simulink model "Final_V2"  (R2024a)
settings: Solver=FixedStepAuto  StartTime=0.0  StopTime=inf  FixedStep=1e-3  RelTol=1e-3
libraries: fl_lib x107, nesl_utility x75, ee_lib x16, batteryecm_lib x14, simulink x2
(part 1 of 16)
settings Model: Creator=DELL  LastModifiedBy=DELL

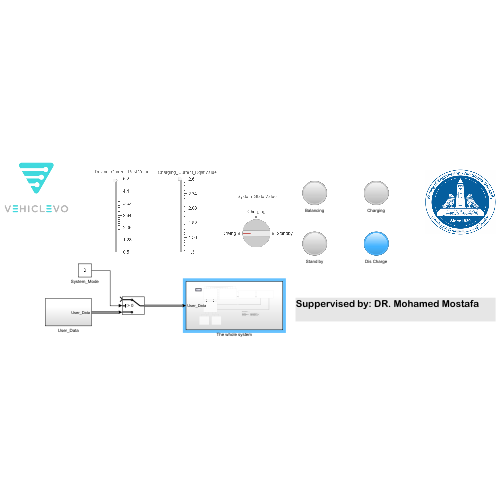
[thumbnail: Simulink's stored preview of the top-level diagram]
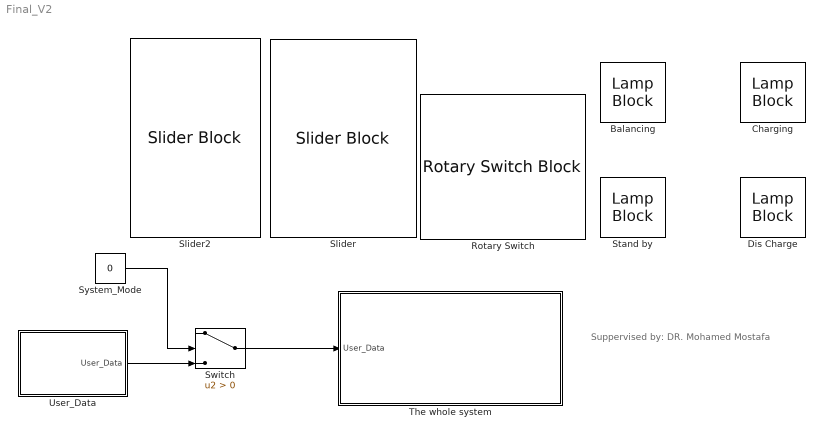
== system Final_V2 ==
  note: Suppervised by: DR. Mohamed Mostafa
  Balancing : LampBlock  LabelPosition=Hide
  Charging : LampBlock  LabelPosition=Hide
  Dis Charge : LampBlock  LabelPosition=Hide
  Rotary Switch : RotarySwitchBlock
      settings: Values={{'Driving', 'Charging', 'Standby'}, [1 2 3]}
  Slider : SliderBlock  ScaleMin=1.3  ScaleMax=2.6
  Slider2 : SliderBlock  ScaleMin=.5  ScaleMax=5.2
  Stand by : LampBlock  LabelPosition=Hide
  Switch : Switch  Criteria=u2 > Threshold  SaturateOnIntegerOverflow=off  InputSameDT=off
  System_Mode : Constant  Value=0
  The whole system : Subsystem
  User_Data : Subsystem
  wires:
  System_Mode/1 -> Switch/2
  User_Data/1(User_Data) -> Switch/3
  Switch/1 -> The whole system/1(User_Data)

== system Final_V2/The whole system ==
  note: Ziad Yasser
      | Ganna Ayman
      | Abdullah Mohamed
      | Amr Ahmed
  note: Youmna Mohamed
      | Ahmed Hassan
      | Abdelrahman Mohamed
      | Khaled Abdelmonem
  note: Team members:
  User_Data : Inport
  Battery Pack : Subsystem  mask{Ambient_Temp=25}
      settings: PortSchema="{\"entries\":[{\"content\":{\"sides\":[{\"content\":{\"connectorIds\":[\"LConn1\",\"LConn2\",\"LConn3\",\"LConn4\",\"LConn5\",\"LConn6\",\"LConn7\",\"LConn8\",\"LConn9\",\"LConn10\",\"LConn11\",\"LConn12\",\"LConn13\",\"LConn14\",\"LConn15\"]},\"type\":\"ConnectorPlacement.EquallySpacedRectSide\",\"uuid\":\"6eeafaf8-a6ff-46ec-b374-5c9558204498\"},{\"content\":{\"side\":\"RIGHT\"},\"type\":\"ConnectorPlacement.EquallySpacedRectSide\",\"uuid\":\"4357267c-e777-417e-805c-0985daf4fb09\"},{\"content\":{\"connectorIds\":[\"Out1\",\"Out2\",\"Out3\",\"Out4\",\"Out5\",\"Out6\",\"Out7\"],\"side\":\"TOP\"},\"type\":\"ConnectorPlacement.EquallySpacedRectSide\",\"uuid\":\"0f9893f0-e258-44a1-af45-4bd291edbfdd\"},{\"content\":{\"connectorIds\":[\"RConn1\",\"RConn2\"],\"side\":\"BOTTOM\"},\"type\":\"ConnectorPlacement.EquallySpacedRectSide\",\"uuid\":\"5ba0f9cc-7562-4c1c-a822-eec2eb98cfa4\"}]},\"type\":\"ConnectorPlacement.EquallySpacedConnector\",\"uuid\":\"b3bd4dec-16f3-46e1-be5d-352b3f05ca49\"}],\"packageUris\":[\"http://schema.mathworks.com/mf0/Connector/R2020b\"],\"version\":\"1.0\"}"
      mask Display: image('battery_pack.jpg');
  Charger : Subsystem
      mask Display: image('charger.jpg');
  Contactor Module : Subsystem
      settings: PortSchema="{\"entries\":[{\"content\":{\"sides\":[{\"content\":{},\"type\":\"ConnectorPlacement.EquallySpacedRectSide\",\"uuid\":\"ee209af3-0e56-4b18-8b81-9599617d44d5\"},{\"content\":{\"connectorIds\":[\"Out1\",\"In1\"],\"side\":\"RIGHT\"},\"type\":\"ConnectorPlacement.EquallySpacedRectSide\",\"uuid\":\"255f66a6-4968-490c-a47d-683fa91efcb4\"},{\"content\":{\"connectorIds\":[\"LConn1\",\"LConn2\"],\"side\":\"TOP\"},\"type\":\"ConnectorPlacement.EquallySpacedRectSide\",\"uuid\":\"e4e998d2-707a-4114-9ad5-887fac7a97e5\"},{\"content\":{\"connectorIds\":[\"RConn1\",\"RConn2\",\"RConn3\",\"RConn4\"],\"side\":\"BOTTOM\"},\"type\":\"ConnectorPlacement.EquallySpacedRectSide\",\"uuid\":\"cb2d048d-e6e1-4998-b640-d93e70266657\"}]},\"type\":\"ConnectorPlacement.EquallySpacedConnector\",\"uuid\":\"827b1e79-e2b6-4c20-843a-a8dc262c0294\"}],\"packageUris\":[\"http://schema.mathworks.com/mf0/Connector/R2020b\"],\"version\":\"1.0\"}"
  Delay1 : Delay  DelayLength=1
  Isolated Network Transceiver : Subsystem
      settings: PortSchema="{\"entries\":[{\"content\":{\"sides\":[{\"content\":{\"connectorIds\":[\"Out2\",\"In1\"]},\"type\":\"ConnectorPlacement.EquallySpacedRectSide\",\"uuid\":\"2af8957d-6a32-4e6a-8614-81683e096fb5\"},{\"content\":{\"connectorIds\":[\"In2\",\"Out1\"],\"side\":\"RIGHT\"},\"type\":\"ConnectorPlacement.EquallySpacedRectSide\",\"uuid\":\"f59defdc-cb0b-4d85-a078-30d88b77abef\"},{\"content\":{\"side\":\"TOP\"},\"type\":\"ConnectorPlacement.EquallySpacedRectSide\",\"uuid\":\"86c096d0-c0e4-46e7-a677-1a376ae83d91\"}]},\"type\":\"ConnectorPlacement.EquallySpacedConnector\",\"uuid\":\"7c251f68-3924-43c9-9cff-d35df31b019f\"}],\"packageUris\":[\"http://schema.mathworks.com/mf0/Connector/R2020b\"],\"version\":\"1.0\"}"
      mask Display: image('FRDM33664BEVB.png');
  Load : Subsystem
      mask Display: image('Load.jpg');
  Master : Subsystem
      settings: PortSchema="{\"entries\":[{\"content\":{\"sides\":[{\"content\":{\"connectorIds\":[\"Out2\",\"In1\"]},\"type\":\"ConnectorPlacement.EquallySpacedRectSide\",\"uuid\":\"2af8957d-6a32-4e6a-8614-81683e096fb5\"},{\"content\":{\"side\":\"RIGHT\"},\"type\":\"ConnectorPlacement.EquallySpacedRectSide\",\"uuid\":\"f59defdc-cb0b-4d85-a078-30d88b77abef\"},{\"content\":{\"side\":\"TOP\"},\"type\":\"ConnectorPlacement.EquallySpacedRectSide\",\"uuid\":\"86c096d0-c0e4-46e7-a677-1a376ae83d91\"},{\"content\":{\"connectorIds\":[\"In2\",\"Out1\"],\"side\":\"BOTTOM\"},\"type\":\"ConnectorPlacement.EquallySpacedRectSide\",\"uuid\":\"98b88255-9217-4b94-835c-9d931802242b\"}]},\"type\":\"ConnectorPlacement.EquallySpacedConnector\",\"uuid\":\"7c251f68-3924-43c9-9cff-d35df31b019f\"}],\"packageUris\":[\"http://schema.mathworks.com/mf0/Connector/R2020b\"],\"version\":\"1.0\"}"
      mask Display: image('FRDM_KL25Z.png');
  SLAVE FRDM33771BTPLEVB : Subsystem
      settings: PortSchema="{\"entries\":[{\"content\":{\"sides\":[{\"content\":{\"connectorIds\":[\"LConn1\",\"LConn2\",\"LConn3\",\"LConn4\",\"LConn5\",\"LConn6\",\"LConn7\",\"LConn8\",\"LConn9\",\"LConn10\",\"LConn11\",\"LConn12\",\"LConn13\",\"LConn14\",\"LConn15\"]},\"type\":\"ConnectorPlacement.EquallySpacedRectSide\",\"uuid\":\"01deb29f-ef65-4542-a3bf-9b8dca36de76\"},{\"content\":{\"connectorIds\":[\"In8\",\"Out1\"],\"side\":\"RIGHT\"},\"type\":\"ConnectorPlacement.EquallySpacedRectSide\",\"uuid\":\"ca53145e-e40a-4aca-9705-ce2867e97f90\"},{\"content\":{\"connectorIds\":[\"In1\",\"In2\",\"In3\",\"In4\",\"In5\",\"In6\",\"In7\"],\"side\":\"TOP\"},\"type\":\"ConnectorPlacement.EquallySpacedRectSide\",\"uuid\":\"cefc55b1-f04c-4c0e-881d-02bf76fe597c\"},{\"content\":{\"side\":\"BOTTOM\"},\"type\":\"ConnectorPlacement.EquallySpacedRectSide\",\"uuid\":\"19ffcb40-a2b6-48de-9ba1-36ba9ec5dcae\"}]},\"type\":\"ConnectorPlacement.EquallySpacedConnector\",\"uuid\":\"5b0a51cf-d63d-4ef3-94c6-6844a83c1e4b\"}],\"packageUris\":[\"http://schema.mathworks.com/mf0/Connector/R2020b\"],\"version\":\"1.0\"}"
  tag scope: User_Data=global
  wires:
  User_Data/1 -> [User_Data]
  Isolated Network Transceiver/2(To_Slave) -> SLAVE FRDM33771BTPLEVB/8(From_Transceiver)
  SLAVE FRDM33771BTPLEVB/1(To_Transceiver) -> Isolated Network Transceiver/1(From_Slave)
  Master/2(To_Transceiver) -> Isolated Network Transceiver/2(From_Master)
  Isolated Network Transceiver/1(To_Master) -> Master/1(From_Transceiver)
  Contactor Module/1(To_Master) -> Delay1/1
  Battery Pack/1(Temp.13&14) -> SLAVE FRDM33771BTPLEVB/1(Temp.13&14)
  Battery Pack/2(Temp.11&12) -> SLAVE FRDM33771BTPLEVB/2(Temp.11&12)
  Battery Pack/3(Temp.9&10) -> SLAVE FRDM33771BTPLEVB/3(Temp.9&10)
  Battery Pack/4(Temp.7&8) -> SLAVE FRDM33771BTPLEVB/4(Temp.7&8)
  Battery Pack/5(Temp.5&6) -> SLAVE FRDM33771BTPLEVB/5(Temp.5&6)
  Battery Pack/6(Temp.3&4) -> SLAVE FRDM33771BTPLEVB/6(Temp.3&4)
  Battery Pack/7(Temp.1&2) -> SLAVE FRDM33771BTPLEVB/7(Temp.1&2)
  Master/1(To_Contactor_Module) -> Contactor Module/1(From_Master)
  Delay1/1 -> Master/2(From_Contactor_Module)
  connections:
  Battery Pack/RConn2 -- Contactor Module/LConn1
  Battery Pack/RConn1 -- Contactor Module/LConn2
  Battery Pack/LConn1 -- SLAVE FRDM33771BTPLEVB/LConn1
  Battery Pack/LConn2 -- SLAVE FRDM33771BTPLEVB/LConn2
  Battery Pack/LConn3 -- SLAVE FRDM33771BTPLEVB/LConn3
  Battery Pack/LConn4 -- SLAVE FRDM33771BTPLEVB/LConn4
  Battery Pack/LConn5 -- SLAVE FRDM33771BTPLEVB/LConn5
  Battery Pack/LConn6 -- SLAVE FRDM33771BTPLEVB/LConn6
  SLAVE FRDM33771BTPLEVB/LConn7 -- Battery Pack/LConn7
  Battery Pack/LConn8 -- SLAVE FRDM33771BTPLEVB/LConn8
  SLAVE FRDM33771BTPLEVB/LConn9 -- Battery Pack/LConn9
  Battery Pack/LConn10 -- SLAVE FRDM33771BTPLEVB/LConn10
  SLAVE FRDM33771BTPLEVB/LConn11 -- Battery Pack/LConn11
  Battery Pack/LConn12 -- SLAVE FRDM33771BTPLEVB/LConn12
  SLAVE FRDM33771BTPLEVB/LConn13 -- Battery Pack/LConn13
  Battery Pack/LConn14 -- SLAVE FRDM33771BTPLEVB/LConn14
  SLAVE FRDM33771BTPLEVB/LConn15 -- Battery Pack/LConn15
  Load/LConn2 -- Contactor Module/RConn3
  Load/LConn1 -- Contactor Module/RConn4
  Charger/LConn2 -- Contactor Module/RConn1
  Charger/LConn1 -- Contactor Module/RConn2

== system Final_V2/User_Data ==
  Bus Creator : BusCreator  Inputs=3
  Bus Creator2 : BusCreator  Inputs=2
  Charging_Current_Rqst : Constant  Value=2.6
  Constant : Constant
  Driving_Current_Rqst : Constant  Value=5.071589108406963
  Driving_Current_Selector : Constant  Value=0
  Switch : Switch  Criteria=u2 > Threshold  SaturateOnIntegerOverflow=off  InputSameDT=off
  System_State : Constant
  User_Data : Outport
  wires:
  Driving_Current_Rqst/1 -> Switch/3
  Driving_Current_Selector/1 -> Switch/2
  System_State/1 -> Bus Creator/1  "System_State"
  Switch/1 -> Bus Creator/2  "Driving_Current_Rqst"
  Charging_Current_Rqst/1 -> Bus Creator/3  "Charging_Current_Rqst"
  Bus Creator/1 -> Bus Creator2/1  "User_State"
  Bus Creator2/1 -> User_Data/1  "User_Data"
  Constant/1 -> Switch/1

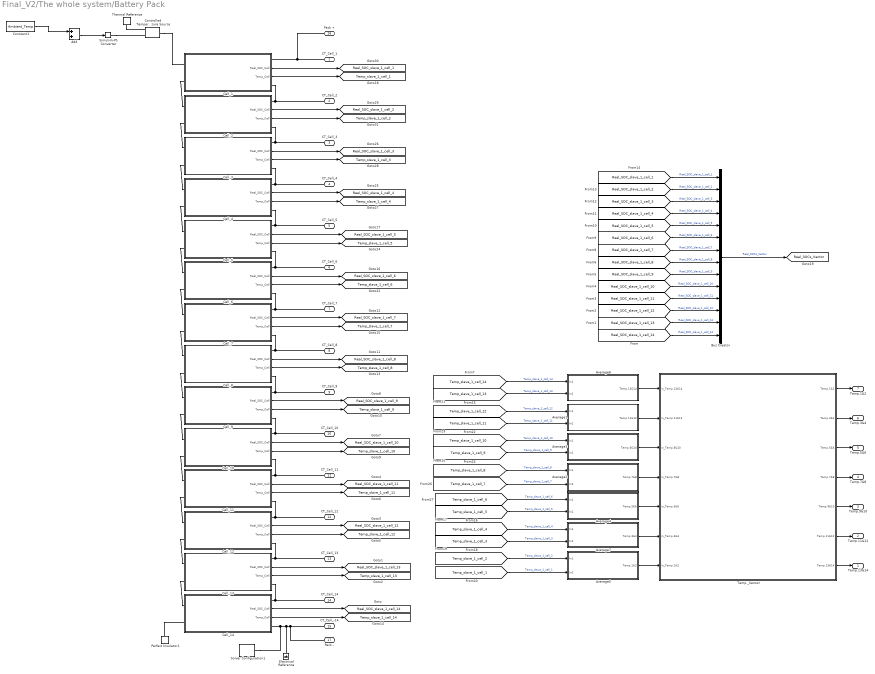
== system Final_V2/The whole system/Battery Pack ==
  Add : Sum
  Average1 : Subsystem
  Average2 : Subsystem
  Average3 : Subsystem
  Average4 : Subsystem
  Average5 : Subsystem
  Average6 : Subsystem
  Average7 : Subsystem
  Bus Creator : BusCreator  Inputs=14
  Cell_1 : Subsystem
      settings: PortSchema="{\"entries\":[{\"content\":{\"sides\":[{\"content\":{\"connectorIds\":[\"LConn1\",\"LConn2\"]},\"type\":\"ConnectorPlacement.EquallySpacedRectSide\",\"uuid\":\"d81198d0-21e5-4c7d-b16f-80ee729df27d\"},{\"content\":{\"connectorIds\":[\"RConn1\",\"Out1\",\"Out2\",\"RConn2\"],\"side\":\"RIGHT\"},\"type\":\"ConnectorPlacement.EquallySpacedRectSide\",\"uuid\":\"649ab37d-8508-4061-b6e0-d2c44b214558\"},{\"content\":{\"side\":\"TOP\"},\"type\":\"ConnectorPlacement.EquallySpacedRectSide\",\"uuid\":\"4eaeca9b-2db5-4b04-8251-969f6802694c\"}]},\"type\":\"ConnectorPlacement.EquallySpacedConnector\",\"uuid\":\"fe913d55-a0ee-44c6-9488-2c5127d79dbf\"}],\"packageUris\":[\"http://schema.mathworks.com/mf0/Connector/R2020b\"],\"version\":\"1.0\"}"
  Cell_10 : Subsystem
      settings: PortSchema="{\"entries\":[{\"content\":{\"sides\":[{\"content\":{\"connectorIds\":[\"LConn1\",\"LConn2\"]},\"type\":\"ConnectorPlacement.EquallySpacedRectSide\",\"uuid\":\"d81198d0-21e5-4c7d-b16f-80ee729df27d\"},{\"content\":{\"connectorIds\":[\"RConn1\",\"Out1\",\"Out2\",\"RConn2\"],\"side\":\"RIGHT\"},\"type\":\"ConnectorPlacement.EquallySpacedRectSide\",\"uuid\":\"649ab37d-8508-4061-b6e0-d2c44b214558\"},{\"content\":{\"side\":\"TOP\"},\"type\":\"ConnectorPlacement.EquallySpacedRectSide\",\"uuid\":\"4eaeca9b-2db5-4b04-8251-969f6802694c\"}]},\"type\":\"ConnectorPlacement.EquallySpacedConnector\",\"uuid\":\"fe913d55-a0ee-44c6-9488-2c5127d79dbf\"}],\"packageUris\":[\"http://schema.mathworks.com/mf0/Connector/R2020b\"],\"version\":\"1.0\"}"
  Cell_11 : Subsystem
      settings: PortSchema="{\"entries\":[{\"content\":{\"sides\":[{\"content\":{\"connectorIds\":[\"LConn1\",\"LConn2\"]},\"type\":\"ConnectorPlacement.EquallySpacedRectSide\",\"uuid\":\"d81198d0-21e5-4c7d-b16f-80ee729df27d\"},{\"content\":{\"connectorIds\":[\"RConn1\",\"Out1\",\"Out2\",\"RConn2\"],\"side\":\"RIGHT\"},\"type\":\"ConnectorPlacement.EquallySpacedRectSide\",\"uuid\":\"649ab37d-8508-4061-b6e0-d2c44b214558\"},{\"content\":{\"side\":\"TOP\"},\"type\":\"ConnectorPlacement.EquallySpacedRectSide\",\"uuid\":\"4eaeca9b-2db5-4b04-8251-969f6802694c\"}]},\"type\":\"ConnectorPlacement.EquallySpacedConnector\",\"uuid\":\"fe913d55-a0ee-44c6-9488-2c5127d79dbf\"}],\"packageUris\":[\"http://schema.mathworks.com/mf0/Connector/R2020b\"],\"version\":\"1.0\"}"
  Cell_12 : Subsystem
      settings: PortSchema="{\"entries\":[{\"content\":{\"sides\":[{\"content\":{\"connectorIds\":[\"LConn1\",\"LConn2\"]},\"type\":\"ConnectorPlacement.EquallySpacedRectSide\",\"uuid\":\"d81198d0-21e5-4c7d-b16f-80ee729df27d\"},{\"content\":{\"connectorIds\":[\"RConn1\",\"Out1\",\"Out2\",\"RConn2\"],\"side\":\"RIGHT\"},\"type\":\"ConnectorPlacement.EquallySpacedRectSide\",\"uuid\":\"649ab37d-8508-4061-b6e0-d2c44b214558\"},{\"content\":{\"side\":\"TOP\"},\"type\":\"ConnectorPlacement.EquallySpacedRectSide\",\"uuid\":\"4eaeca9b-2db5-4b04-8251-969f6802694c\"}]},\"type\":\"ConnectorPlacement.EquallySpacedConnector\",\"uuid\":\"fe913d55-a0ee-44c6-9488-2c5127d79dbf\"}],\"packageUris\":[\"http://schema.mathworks.com/mf0/Connector/R2020b\"],\"version\":\"1.0\"}"
  Cell_13 : Subsystem
      settings: PortSchema="{\"entries\":[{\"content\":{\"sides\":[{\"content\":{\"connectorIds\":[\"LConn1\",\"LConn2\"]},\"type\":\"ConnectorPlacement.EquallySpacedRectSide\",\"uuid\":\"d81198d0-21e5-4c7d-b16f-80ee729df27d\"},{\"content\":{\"connectorIds\":[\"RConn1\",\"Out1\",\"Out2\",\"RConn2\"],\"side\":\"RIGHT\"},\"type\":\"ConnectorPlacement.EquallySpacedRectSide\",\"uuid\":\"649ab37d-8508-4061-b6e0-d2c44b214558\"},{\"content\":{\"side\":\"TOP\"},\"type\":\"ConnectorPlacement.EquallySpacedRectSide\",\"uuid\":\"4eaeca9b-2db5-4b04-8251-969f6802694c\"}]},\"type\":\"ConnectorPlacement.EquallySpacedConnector\",\"uuid\":\"fe913d55-a0ee-44c6-9488-2c5127d79dbf\"}],\"packageUris\":[\"http://schema.mathworks.com/mf0/Connector/R2020b\"],\"version\":\"1.0\"}"
  Cell_14 : Subsystem
      settings: PortSchema="{\"entries\":[{\"content\":{\"sides\":[{\"content\":{\"connectorIds\":[\"LConn1\",\"LConn2\"]},\"type\":\"ConnectorPlacement.EquallySpacedRectSide\",\"uuid\":\"d81198d0-21e5-4c7d-b16f-80ee729df27d\"},{\"content\":{\"connectorIds\":[\"RConn1\",\"Out1\",\"Out2\",\"RConn2\"],\"side\":\"RIGHT\"},\"type\":\"ConnectorPlacement.EquallySpacedRectSide\",\"uuid\":\"649ab37d-8508-4061-b6e0-d2c44b214558\"},{\"content\":{\"side\":\"TOP\"},\"type\":\"ConnectorPlacement.EquallySpacedRectSide\",\"uuid\":\"4eaeca9b-2db5-4b04-8251-969f6802694c\"}]},\"type\":\"ConnectorPlacement.EquallySpacedConnector\",\"uuid\":\"fe913d55-a0ee-44c6-9488-2c5127d79dbf\"}],\"packageUris\":[\"http://schema.mathworks.com/mf0/Connector/R2020b\"],\"version\":\"1.0\"}"
  Cell_2 : Subsystem
      settings: PortSchema="{\"entries\":[{\"content\":{\"sides\":[{\"content\":{\"connectorIds\":[\"LConn1\",\"LConn2\"]},\"type\":\"ConnectorPlacement.EquallySpacedRectSide\",\"uuid\":\"d81198d0-21e5-4c7d-b16f-80ee729df27d\"},{\"content\":{\"connectorIds\":[\"RConn1\",\"Out1\",\"Out2\",\"RConn2\"],\"side\":\"RIGHT\"},\"type\":\"ConnectorPlacement.EquallySpacedRectSide\",\"uuid\":\"649ab37d-8508-4061-b6e0-d2c44b214558\"},{\"content\":{\"side\":\"TOP\"},\"type\":\"ConnectorPlacement.EquallySpacedRectSide\",\"uuid\":\"4eaeca9b-2db5-4b04-8251-969f6802694c\"}]},\"type\":\"ConnectorPlacement.EquallySpacedConnector\",\"uuid\":\"fe913d55-a0ee-44c6-9488-2c5127d79dbf\"}],\"packageUris\":[\"http://schema.mathworks.com/mf0/Connector/R2020b\"],\"version\":\"1.0\"}"
  Cell_3 : Subsystem
      settings: PortSchema="{\"entries\":[{\"content\":{\"sides\":[{\"content\":{\"connectorIds\":[\"LConn1\",\"LConn2\"]},\"type\":\"ConnectorPlacement.EquallySpacedRectSide\",\"uuid\":\"d81198d0-21e5-4c7d-b16f-80ee729df27d\"},{\"content\":{\"connectorIds\":[\"RConn1\",\"Out1\",\"Out2\",\"RConn2\"],\"side\":\"RIGHT\"},\"type\":\"ConnectorPlacement.EquallySpacedRectSide\",\"uuid\":\"649ab37d-8508-4061-b6e0-d2c44b214558\"},{\"content\":{\"side\":\"TOP\"},\"type\":\"ConnectorPlacement.EquallySpacedRectSide\",\"uuid\":\"4eaeca9b-2db5-4b04-8251-969f6802694c\"}]},\"type\":\"ConnectorPlacement.EquallySpacedConnector\",\"uuid\":\"fe913d55-a0ee-44c6-9488-2c5127d79dbf\"}],\"packageUris\":[\"http://schema.mathworks.com/mf0/Connector/R2020b\"],\"version\":\"1.0\"}"
  Cell_4 : Subsystem
      settings: PortSchema="{\"entries\":[{\"content\":{\"sides\":[{\"content\":{\"connectorIds\":[\"LConn1\",\"LConn2\"]},\"type\":\"ConnectorPlacement.EquallySpacedRectSide\",\"uuid\":\"d81198d0-21e5-4c7d-b16f-80ee729df27d\"},{\"content\":{\"connectorIds\":[\"RConn1\",\"Out1\",\"Out2\",\"RConn2\"],\"side\":\"RIGHT\"},\"type\":\"ConnectorPlacement.EquallySpacedRectSide\",\"uuid\":\"649ab37d-8508-4061-b6e0-d2c44b214558\"},{\"content\":{\"side\":\"TOP\"},\"type\":\"ConnectorPlacement.EquallySpacedRectSide\",\"uuid\":\"4eaeca9b-2db5-4b04-8251-969f6802694c\"}]},\"type\":\"ConnectorPlacement.EquallySpacedConnector\",\"uuid\":\"fe913d55-a0ee-44c6-9488-2c5127d79dbf\"}],\"packageUris\":[\"http://schema.mathworks.com/mf0/Connector/R2020b\"],\"version\":\"1.0\"}"
  Cell_5 : Subsystem
      settings: PortSchema="{\"entries\":[{\"content\":{\"sides\":[{\"content\":{\"connectorIds\":[\"LConn1\",\"LConn2\"]},\"type\":\"ConnectorPlacement.EquallySpacedRectSide\",\"uuid\":\"d81198d0-21e5-4c7d-b16f-80ee729df27d\"},{\"content\":{\"connectorIds\":[\"RConn1\",\"Out1\",\"Out2\",\"RConn2\"],\"side\":\"RIGHT\"},\"type\":\"ConnectorPlacement.EquallySpacedRectSide\",\"uuid\":\"649ab37d-8508-4061-b6e0-d2c44b214558\"},{\"content\":{\"side\":\"TOP\"},\"type\":\"ConnectorPlacement.EquallySpacedRectSide\",\"uuid\":\"4eaeca9b-2db5-4b04-8251-969f6802694c\"}]},\"type\":\"ConnectorPlacement.EquallySpacedConnector\",\"uuid\":\"fe913d55-a0ee-44c6-9488-2c5127d79dbf\"}],\"packageUris\":[\"http://schema.mathworks.com/mf0/Connector/R2020b\"],\"version\":\"1.0\"}"
  Cell_6 : Subsystem
      settings: PortSchema="{\"entries\":[{\"content\":{\"sides\":[{\"content\":{\"connectorIds\":[\"LConn1\",\"LConn2\"]},\"type\":\"ConnectorPlacement.EquallySpacedRectSide\",\"uuid\":\"d81198d0-21e5-4c7d-b16f-80ee729df27d\"},{\"content\":{\"connectorIds\":[\"RConn1\",\"Out1\",\"Out2\",\"RConn2\"],\"side\":\"RIGHT\"},\"type\":\"ConnectorPlacement.EquallySpacedRectSide\",\"uuid\":\"649ab37d-8508-4061-b6e0-d2c44b214558\"},{\"content\":{\"side\":\"TOP\"},\"type\":\"ConnectorPlacement.EquallySpacedRectSide\",\"uuid\":\"4eaeca9b-2db5-4b04-8251-969f6802694c\"}]},\"type\":\"ConnectorPlacement.EquallySpacedConnector\",\"uuid\":\"fe913d55-a0ee-44c6-9488-2c5127d79dbf\"}],\"packageUris\":[\"http://schema.mathworks.com/mf0/Connector/R2020b\"],\"version\":\"1.0\"}"
  Cell_7 : Subsystem
      settings: PortSchema="{\"entries\":[{\"content\":{\"sides\":[{\"content\":{\"connectorIds\":[\"LConn1\",\"LConn2\"]},\"type\":\"ConnectorPlacement.EquallySpacedRectSide\",\"uuid\":\"d81198d0-21e5-4c7d-b16f-80ee729df27d\"},{\"content\":{\"connectorIds\":[\"RConn1\",\"Out1\",\"Out2\",\"RConn2\"],\"side\":\"RIGHT\"},\"type\":\"ConnectorPlacement.EquallySpacedRectSide\",\"uuid\":\"649ab37d-8508-4061-b6e0-d2c44b214558\"},{\"content\":{\"side\":\"TOP\"},\"type\":\"ConnectorPlacement.EquallySpacedRectSide\",\"uuid\":\"4eaeca9b-2db5-4b04-8251-969f6802694c\"}]},\"type\":\"ConnectorPlacement.EquallySpacedConnector\",\"uuid\":\"fe913d55-a0ee-44c6-9488-2c5127d79dbf\"}],\"packageUris\":[\"http://schema.mathworks.com/mf0/Connector/R2020b\"],\"version\":\"1.0\"}"
  Cell_8 : Subsystem
      settings: PortSchema="{\"entries\":[{\"content\":{\"sides\":[{\"content\":{\"connectorIds\":[\"LConn1\",\"LConn2\"]},\"type\":\"ConnectorPlacement.EquallySpacedRectSide\",\"uuid\":\"d81198d0-21e5-4c7d-b16f-80ee729df27d\"},{\"content\":{\"connectorIds\":[\"RConn1\",\"Out1\",\"Out2\",\"RConn2\"],\"side\":\"RIGHT\"},\"type\":\"ConnectorPlacement.EquallySpacedRectSide\",\"uuid\":\"649ab37d-8508-4061-b6e0-d2c44b214558\"},{\"content\":{\"side\":\"TOP\"},\"type\":\"ConnectorPlacement.EquallySpacedRectSide\",\"uuid\":\"4eaeca9b-2db5-4b04-8251-969f6802694c\"}]},\"type\":\"ConnectorPlacement.EquallySpacedConnector\",\"uuid\":\"fe913d55-a0ee-44c6-9488-2c5127d79dbf\"}],\"packageUris\":[\"http://schema.mathworks.com/mf0/Connector/R2020b\"],\"version\":\"1.0\"}"
  Cell_9 : Subsystem
      settings: PortSchema="{\"entries\":[{\"content\":{\"sides\":[{\"content\":{\"connectorIds\":[\"LConn1\",\"LConn2\"]},\"type\":\"ConnectorPlacement.EquallySpacedRectSide\",\"uuid\":\"d81198d0-21e5-4c7d-b16f-80ee729df27d\"},{\"content\":{\"connectorIds\":[\"RConn1\",\"Out1\",\"Out2\",\"RConn2\"],\"side\":\"RIGHT\"},\"type\":\"ConnectorPlacement.EquallySpacedRectSide\",\"uuid\":\"649ab37d-8508-4061-b6e0-d2c44b214558\"},{\"content\":{\"side\":\"TOP\"},\"type\":\"ConnectorPlacement.EquallySpacedRectSide\",\"uuid\":\"4eaeca9b-2db5-4b04-8251-969f6802694c\"}]},\"type\":\"ConnectorPlacement.EquallySpacedConnector\",\"uuid\":\"fe913d55-a0ee-44c6-9488-2c5127d79dbf\"}],\"packageUris\":[\"http://schema.mathworks.com/mf0/Connector/R2020b\"],\"version\":\"1.0\"}"
  Constant1 : Constant  Value=Ambient_Temp
  Controlled Temperature Source : fl_lib/Thermal/Thermal Sources/Controlled Temperature Source
  Electrical Reference : fl_lib/Electrical/Electrical Elements/Electrical Reference
  Perfect Insulator1 : fl_lib/Thermal/Thermal Elements/Perfect Insulator  T_specify=off  T_priority=None  T=293.15 K  T_nominal_specify=off  T_nominal_value=1  T_nominal_unit=K
  Simulink-PS Converter : nesl_utility/Simulink-PS Converter  PhysicalDomain=network_engine_domain  SubClassName=ps_input  LeftPortType=input  RightPortType=output  PseudoPeriodic=off  Frequencies=[]  NoiseDistribution=none  NoiseParameters=[]  Unit=1  AffineConversion=off  FilteringAndDerivatives=provide  SimscapeFilterOrder=1  InputFilterTimeConstant=0.001  UdotUserProvided=0
  Solver Configuration1 : nesl_utility/Solver Configuration  PhysicalDomain=network_engine_domain  SubClassName=solver  LeftPortType=input  RightPortType=generic  Tones=[]  Harmonics=[]  SimulateNoise=off  Profile=off  UseLocalSampling=off  EnableSwitchedLinearOptims=on  FrequencyDomain=off  UseCCode=off  RelTol=0.001  AbsTol=0.001  MinStep=1e-09  ToWorkspace=off  RevertToSquareIcSolve=off  NumHomotopyIterations=100  PhasorMode=off  PhasorModeNumHarmonics=1  ConsistencySolver=NEWTON_XTOL_AFTER_FTOL  IndexReductionMethod=DerivativeReplacement  DoDC=off  ResidualTolerance=1e-09  ConsistencyTolSource=GLOBAL  ConsistencyAbsTol=0.001  ConsistencyRelTol=0.001  ConsistencyTolFactor=0.001  UseLocalSolver=off  LocalSolverChoice=NE_BACKWARD_EULER_ADVANCER  ResolveIndetEquations=on  FunctionEvalNumThread=1  LocalSolverSampleTime=0.001  DoFixedCost=off  MaxNonlinIter=3  MaxModeIter=2  ComputeImpulses=off  ImpulseIterations=2  LinearAlgebra=auto  LinearAlgebraNumThread=0  EquationFormulation=NE_TIME_EF  DelaysMemoryBudget=1024  AutomaticFiltering=on  FilteringTimeConstant=0.001  PartitionStorageMethod=AS_NEEDED  PartitionMemoryBudget=1024  PartitionMethod=ROBUST  MultibodyUseLocalSolver=off  MultibodyLocalSolverChoice=ODE1  MultibodyLocalSolverSampleTime=0.001
  Temp._Sensor : Subsystem
  Thermal Reference : fl_lib/Thermal/Thermal Elements/Thermal Reference
  CT_Cell_1 : PMIOPort  Side=Left
  CT_Cell_2 : PMIOPort  Port=2  Side=Left
  CT_Cell_3 : PMIOPort  Port=3  Side=Left
  CT_Cell_4 : PMIOPort  Port=4  Side=Left
  CT_Cell_5 : PMIOPort  Port=5  Side=Left
  CT_Cell_6 : PMIOPort  Port=6  Side=Left
  CT_Cell_7 : PMIOPort  Port=7  Side=Left
  CT_Cell_8 : PMIOPort  Port=8  Side=Left
  CT_Cell_9 : PMIOPort  Port=9  Side=Left
  CT_Cell_10 : PMIOPort  Port=10  Side=Left
  CT_Cell_11 : PMIOPort  Port=11  Side=Left
  CT_Cell_12 : PMIOPort  Port=12  Side=Left
  CT_Cell_13 : PMIOPort  Port=13  Side=Left
  CT_Cell_14 : PMIOPort  Port=14  Side=Left
  CT_Cell_-14 : PMIOPort  Port=15  Side=Left
  Pack + : PMIOPort  Port=16  Side=Right
  Pack - : PMIOPort  Port=17  Side=Right
  Temp.13&14 : Outport
  Temp.11&12 : Outport  Port=2
  Temp.9&10 : Outport  Port=3
  Temp.7&8 : Outport  Port=4
  Temp.5&6 : Outport  Port=5
  Temp.3&4 : Outport  Port=6
  Temp.1&2 : Outport  Port=7
  tag scope: Real_SOC_slave_1_cell_10=global, Real_SOC_slave_1_cell_11=global, Real_SOC_slave_1_cell_12=global, Real_SOC_slave_1_cell_13=global, Real_SOC_slave_1_cell_14=global, Real_SOC_slave_1_cell_1=global, Real_SOC_slave_1_cell_2=global, Real_SOC_slave_1_cell_3=global, Real_SOC_slave_1_cell_4=global, Real_SOC_slave_1_cell_5=global, Real_SOC_slave_1_cell_6=global, Real_SOC_slave_1_cell_7=global, Real_SOC_slave_1_cell_8=global, Real_SOC_slave_1_cell_9=global, Real_SOCs_Vector=global, Temp_slave_1_cell_10=global, Temp_slave_1_cell_11=global, Temp_slave_1_cell_12=global, Temp_slave_1_cell_13=global, Temp_slave_1_cell_14=global, Temp_slave_1_cell_1=global, Temp_slave_1_cell_2=global, Temp_slave_1_cell_3=global, Temp_slave_1_cell_4=global, Temp_slave_1_cell_5=global, Temp_slave_1_cell_6=global, Temp_slave_1_cell_7=global, Temp_slave_1_cell_8=global, Temp_slave_1_cell_9=global